simulink model "CCMMotor"  (R2022b)
settings: Solver=FixedStepDiscrete  StartTime=0.0  StopTime=10.0  FixedStep=0.01  RelTol=1e-3
undefined symbols (not defined in the file or in its folder): DcChlType, HFAChlType, MtrRoutStaEnum, P_Std_ReturnType, VGAChlType
(part 4 of 4)

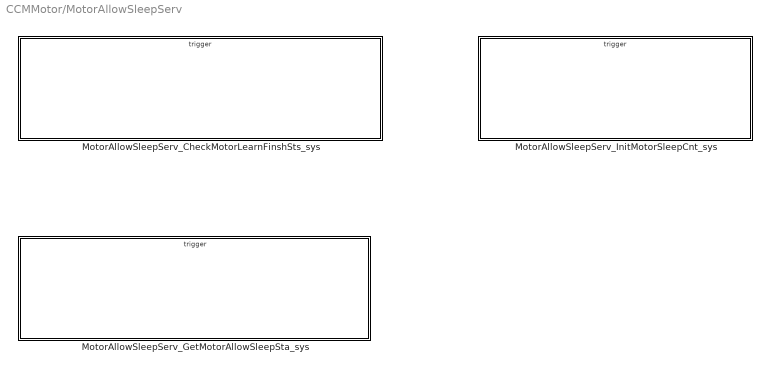
== system CCMMotor/MotorAllowSleepServ ==
  MotorAllowSleepServ_CheckMotorLearnFinshSts_sys : Subsystem
  MotorAllowSleepServ_GetMotorAllowSleepSta_sys : Subsystem
  MotorAllowSleepServ_InitMotorSleepCnt_sys : Subsystem

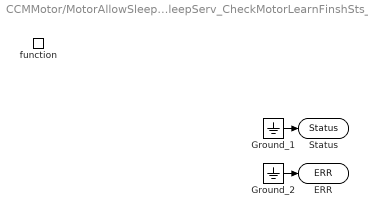
== system CCMMotor/MotorAllowSleepServ/MotorAllowSleepServ_CheckMotorLearnFinshSts_sys ==
  function : TriggerPort  TriggerType=function-call  IsSimulinkFunction=on  FunctionName=MotorAllowSleepServ_CheckMotorLearnFinshSts  VariantControl=(inherit)
  Ground_1 : Ground
  Ground_2 : Ground
  Status : ArgOut  OutDataTypeStr=UInt8  ArgumentName=Status
  ERR : ArgOut  Port=2  OutDataTypeStr=Std_ReturnType  ArgumentName=ERR
  wires:
  Ground_1/1 -> Status/1
  Ground_2/1 -> ERR/1

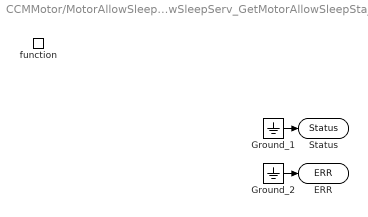
== system CCMMotor/MotorAllowSleepServ/MotorAllowSleepServ_GetMotorAllowSleepSta_sys ==
  function : TriggerPort  TriggerType=function-call  IsSimulinkFunction=on  FunctionName=MotorAllowSleepServ_GetMotorAllowSleepSta  VariantControl=(inherit)
  Ground_1 : Ground
  Ground_2 : Ground
  Status : ArgOut  OutDataTypeStr=UInt8  ArgumentName=Status
  ERR : ArgOut  Port=2  OutDataTypeStr=Std_ReturnType  ArgumentName=ERR
  wires:
  Ground_1/1 -> Status/1
  Ground_2/1 -> ERR/1

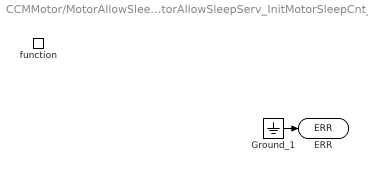
== system CCMMotor/MotorAllowSleepServ/MotorAllowSleepServ_InitMotorSleepCnt_sys ==
  function : TriggerPort  TriggerType=function-call  IsSimulinkFunction=on  FunctionName=MotorAllowSleepServ_InitMotorSleepCnt  VariantControl=(inherit)
  Ground_1 : Ground
  ERR : ArgOut  OutDataTypeStr=Std_ReturnType  ArgumentName=ERR
  wires:
  Ground_1/1 -> ERR/1
